simulink model "dbe_xaui"  (Simulink 7.0)
settings: Solver=ode45  StartTime=0.0  StopTime=1000  RelTol=1e-3
undefined symbols (not defined in the file or in its folder): iBOB, pfb_coeff_gen_calc
libraries: xbsIndex_r4 x1734, casper_library x124, xps_library x28, xbsReplacements_r4 x15, vlbi_lib x5, simulink x4, gavrt_library x1
(part 2 of 17)

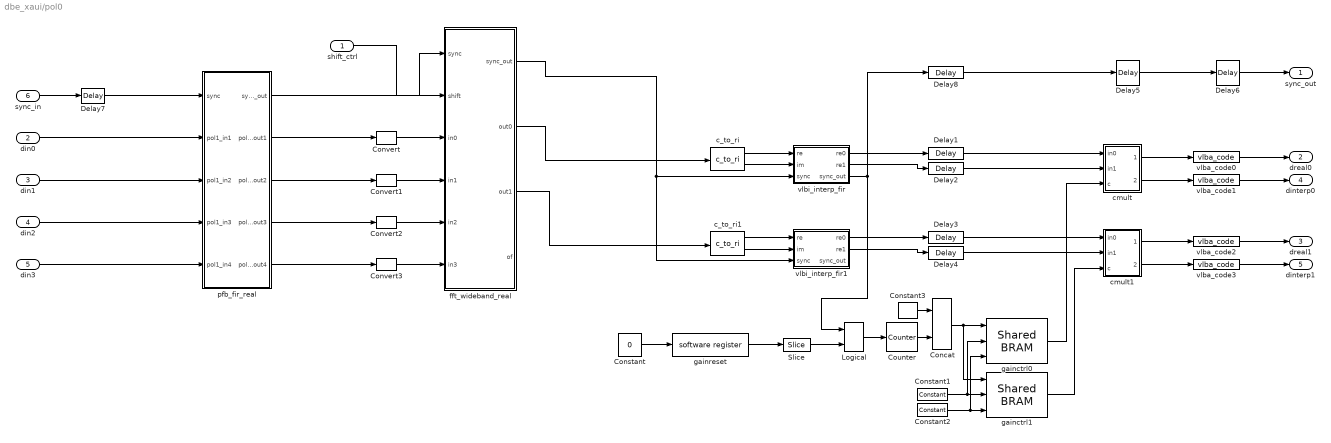
== system dbe_xaui/pol0 ==
  shift_ctrl : Inport  OutDataType=sfix(16)  OutScaling=2^0
  din0 : Inport  Port=2  OutDataType=sfix(16)  OutScaling=2^0
  din1 : Inport  Port=3  OutDataType=sfix(16)  OutScaling=2^0
  din2 : Inport  Port=4  OutDataType=sfix(16)  OutScaling=2^0
  din3 : Inport  Port=5  OutDataType=sfix(16)  OutScaling=2^0
  sync_in : Inport  Port=6  OutDataType=sfix(16)  OutScaling=2^0
  Concat : xbsIndex_r4/Concat  num_inputs=2  dbl_ovrd=off  block_type=concat
      settings: block_version=11.3
  Constant : Constant  Value=0  OutDataType=sfix(16)  OutScaling=2^0
  Constant1 : xbsIndex_r4/Constant  arith_type=Unsigned  const=0  n_bits=32  bin_pt=0  explicit_period=on  period=1  equ=P=C  opselect=C  inp2=PCIN>>17  opr=+  inp1=P  carry=CIN  dbl_ovrd=off  block_type=constant
      settings: block_version=11.3
  Constant2 : xbsIndex_r4/Constant  arith_type=Boolean  const=0  n_bits=1  bin_pt=0  explicit_period=on  period=1  equ=P=C  opselect=C  inp2=PCIN>>17  opr=+  inp1=P  carry=CIN  dbl_ovrd=off  block_type=constant
      settings: block_version=11.3
  Constant3 : xbsIndex_r4/Constant  arith_type=Unsigned  const=0  n_bits=8  bin_pt=0  explicit_period=on  period=1  equ=P=C  opselect=C  inp2=PCIN>>17  opr=+  inp1=P  carry=CIN  dbl_ovrd=off  block_type=constant
      settings: block_version=11.3
  Convert{,1..3} (4 alike) : xbsIndex_r4/Convert  arith_type="Signed  (2's comp)"  n_bits=18  bin_pt=17  quantization=Truncate  overflow=Wrap  latency=0  dbl_ovrd=off  pipeline=off  block_type=convert
      settings: xl_area=[0, 0, 0, 0, 0, 0, 0]  block_version=11.3
  Counter : xbsIndex_r4/Counter  cnt_type=Free Running  cnt_to=Inf  operation=Up  start_count=0  cnt_by_val=1  arith_type=Unsigned  n_bits=3  bin_pt=0  load_pin=off  rst=on  en=off  explicit_period=off  period=1  dbl_ovrd=off  use_behavioral_HDL=off  use_rpm=on  implementation=Fabric  block_type=counter
      settings: xl_area=[0, 0, 0, 0, 0, 0, 0]  block_version=11.3
  Delay1 : xbsIndex_r4/Delay  en=off  latency=1  dbl_ovrd=off  reg_retiming=off  block_type=delay
      settings: xl_area=[0, 0, 0, 0, 0, 0, 0]  block_version=11.3
  Delay{2..4,8} (4 alike) : xbsIndex_r4/Delay  en=off  latency=1  dbl_ovrd=off  reg_retiming=on  block_type=delay
      settings: xl_area=[0, 0, 0, 0, 0, 0, 0]  block_version=11.3
  Delay5 : xbsIndex_r4/Delay  en=off  latency=5  dbl_ovrd=off  reg_retiming=off  block_type=delay
      settings: xl_area=[0, 0, 0, 0, 0, 0, 0]  block_version=11.3
  Delay6 : xbsIndex_r4/Delay  en=off  latency=6  dbl_ovrd=off  reg_retiming=off  block_type=delay
      settings: xl_area=[0, 0, 0, 0, 0, 0, 0]  block_version=11.3
  Delay7 : xbsIndex_r4/Delay  en=off  latency=1  dbl_ovrd=off  reg_retiming=off  block_type=delay
      settings: xl_area=[0, 0, 0, 0, 0, 0, 0]  block_version=11.3
  Logical : xbsIndex_r4/Logical  logical_function=OR  inputs=2  en=off  latency=0  precision=Full  arith_type=Unsigned  n_bits=8  bin_pt=2  align_bp=on  dbl_ovrd=off  block_type=logical
      settings: xl_area=[0, 0, 0, 0, 0, 0, 0]  block_version=11.3
  Slice : xbsIndex_r4/Slice  nbits=1  boolean_output=on  mode=Lower Bit Location + Width  bit1=0  base1=MSB of Input  bit0=0  base0=LSB of Input  dbl_ovrd=off  block_type=slice
      settings: block_version=11.3
  c_to_ri : casper_library/Misc/c_to_ri  SystemSampleTime=-1  n_bits=18  bin_pt=17
      settings: AttributesFormatString=18_17 r/i
  c_to_ri1 : casper_library/Misc/c_to_ri  SystemSampleTime=-1  n_bits=18  bin_pt=17
      settings: AttributesFormatString=18_17 r/i
  cmult : Subsystem  mask{n_bits=18, mult_latency=3}
      mask prompts: n_bits "Bit Width"; mult_latency "Mult Latency"
  cmult1 : Subsystem  mask{n_bits=18, mult_latency=3}
      mask prompts: n_bits "Bit Width"; mult_latency "Mult Latency"
  fft_wideband_real : Subsystem  AncestorBlock=casper_library/FFTs/fft_wideband_real  mask(fft_wideband_real){FFTSize=5, input_bit_width=18, coeff_bit_width=18, n_inputs=2, quantization=Truncate, overflow=Wrap, add_latency=2, mult_latency=3, bram_latency=2, arch=Virtex5, opt_target=Multipliers, coeffs_bit_limit=8, delays_bit_limit=9, specify_mult=off, mult_spec=[2 2 2 2 2], dsp48_adders=off}
      mask prompts: FFTSize "Size of FFT: (2^?)"; input_bit_width "Input/Output Bit Width"; coeff_bit_width "Coefficient Bit Width"; n_inputs "Number of Simultaneous Inputs: (2^?)"; quantization "Quantization Behavior"; overflow "Overflow Behavior"; add_latency "Add Latency"; mult_latency "Mult Latency"; bram_latency "BRAM Latency"; arch "Architecture"; opt_target "Optimisation Target"; coeffs_bit_limit "Number bits above which to store stage's coefficients in BRAM (2^? bits)"; delays_bit_limit "Number bits above which to store stage's delays in BRAM (2^? bits)"; specify_mult "Specify multiplier use (behavioral HDL if not specified)"; mult_spec "Multiplier specification (0=core, 1=embedded, 2=behavioural) (left=1st stage)"; dsp48_adders "Use DSP48s for adders"
      mask init:
      | fft_wideband_real_init(gcb,...
      |     'FFTSize', FFTSize,...
      |     'input_bit_width', input_bit_width,...
      |     'coeff_bit_width', coeff_bit_width,...
      |     'n_inputs', n_inputs,...
      |     'quantization', quantization,...
      |     'overflow', overflow,...
      |     'add_latency', add_latency,...
      |     'mult_latency', mult_latency,...
      |     'bram_latency', bram_latency,...
      |     'arch', arch, ...
      |     'opt_target', opt_target, ...
      |     'coeffs_bit_limit', coeffs_bit_limit, ...
      |     'delays_bit_limit', delays_bit_limit, ...    
      |     'specify_mult', specify_mult,...
      |     'mult_spec', mult_spec, ...
      |     'dsp48_adders', dsp48_adders);
      settings: AttributesFormatString=FFTSize=5
      mask Help: eval('xlWeb([getenv(''MLIB_ROOT''), ''\doc\htmlblocks\sp_fft_wideband_real\sp_fft_wideband_real.html''])')
      mask StyleString: edit,edit,edit,edit,popup(Truncate|Round  (unbiased: +/- Inf)|Round  (unbiased: Even Values)),popup(Wrap|Saturate|Error),edit,edit,edit,popup(Virtex2Pro|Virtex5),popup(Logic|Multipliers),edit,edit,checkbox,edit,checkbox
      mask CallbackString:
      | % fft_size = eval(get_param(gcb, 'FFTSize'));
      | % old_vec = eval(get_param(gcb, 'mult_spec'));
      | 
      | % vec = 2.*ones(1,fft_size);
      | % old_size = length(old_vec);
      | 
      | % if(old_size <= fft_size),
      | %    size = old_size;
      | % else,
      | %     size = fft_size;
      | % end
      | 
      | % for n = 1:size, vec(n) = old_vec(n); end
      | % set_param(gcb, 'mult_spec', mat2str(vec));|||||||||||||specify_mult = get_param(gcb,'specify_mult');
      | visibs = get_param(gcb, 'MaskVisibilities');
      | enables = get_param(gcb, 'MaskEnables');
      | enables{15} = 'on';
      | if( strcmp(specify_mult,'off')), visibs{15} = 'off';
      | else, visibs{15} = 'on';
      | end
      | set_param(gcb, 'MaskEnables', enables);
      | set_param(gcb, 'MaskVisibilities', visibs);
      | |% vec = eval( get_param(gcb, 'mult_spec'));
      | % vec_size = length(vec);
      | % fft_size = eval( get_param(gcb, 'FFTSize'));
      | % new_vec = 2.*ones(1, fft_size);
      | 
      | % if(vec_size <= fft_size), size = vec_size;
      | % else size = fft_size;
      | % end
      | 
      | % for n=1:size
      | %     new_vec(n) = vec(n);
      | % end
      | % set_param(gcb, 'mult_spec', mat2str(new_vec));|
  gainctrl0 : xps_library/Shared BRAM  Tag=xps:bram  SystemSampleTime=-1  arith_type="Signed  (2's comp)"  addr_width=11  data_width=32  data_bin_pt=0  init_vals=[0 0 100 0 zeros(1,2^11-4)]  sample_rate=1
      link override convert_din: arith_type="Signed  (2's comp)"
      link override dbe_xaui_pol0_gainctrl0_data_out: arith_type="Signed  (2's comp)"
      link override mem/sim_data_in: arith_type="Signed  (2's comp)"
      link override vlbipfb2_m3_ibob_pol0_gainctrl0_data_out: arith_type="Signed  (2's comp)"
      link override vlbipfb2_m3_roach_pol0_gainctrl0_data_out: arith_type="Signed  (2's comp)"
  gainctrl1 : xps_library/Shared BRAM  Tag=xps:bram  SystemSampleTime=-1  arith_type="Signed  (2's comp)"  addr_width=11  data_width=32  data_bin_pt=0  init_vals=zeros(1,2^11)  sample_rate=1
      link override convert_din: arith_type="Signed  (2's comp)"
      link override dbe_xaui_pol0_gainctrl1_data_out: arith_type="Signed  (2's comp)"
      link override mem/sim_data_in: arith_type="Signed  (2's comp)"
      link override vlbipfb2_m3_ibob_pol0_gainctrl1_data_out: arith_type="Signed  (2's comp)"
      link override vlbipfb2_m3_roach_pol0_gainctrl1_data_out: arith_type="Signed  (2's comp)"
  gainreset : xps_library/software register  Tag=xps:sw_reg  SystemSampleTime=-1  io_dir=From Processor  arith_type=Unsigned  bitwidth=32  bin_pt=0  sample_period=1  gw_name=dbe_xaui_pol0_gainreset_user_data_out
      link override dbe_xaui_pol0_gainreset_user_data_out: arith_type=Unsigned  n_bits=32  bin_pt=0  period=1
      link override vlbipfb2_m3_ibob_pol0_gainreset_user_data_out: arith_type=Unsigned  n_bits=32  bin_pt=0  period=1
      link override vlbipfb2_m3_roach_pol0_gainreset_user_data_out: arith_type=Unsigned  n_bits=32  bin_pt=0  period=1
  pfb_fir_real : Subsystem  AncestorBlock=casper_library/PFBs/pfb_fir_real  mask(pfb_fir_real){PFBSize=5, TotalTaps=8, WindowType=hamming, n_inputs=2, MakeBiplex=0, BitWidthIn=8, BitWidthOut=8, CoeffBitWidth=8, CoeffDistMem=0, add_latency=2, mult_latency=3, bram_latency=2, quantization=Truncate, fwidth=1, specify_mult=off, mult_spec=[0 1 2 0 1 1 1 1]}
      mask prompts: PFBSize "Size of PFB: (2^? pnts)"; TotalTaps "Total Number of Taps:"; WindowType "Windowing Function:"; n_inputs "Number of Simultaneous Inputs: (2^?)"; MakeBiplex "Make Biplex"; BitWidthIn "Input Bitwidth:"; BitWidthOut "Output Bitwidth:"; CoeffBitWidth "Coefficient Bitwidth:"; CoeffDistMem "Use Distributed Memory for Coeffs"; add_latency "Add Latency"; mult_latency "Mult Latency"; bram_latency "BRAM Latency"; quantization "Quantization Behavior"; fwidth "Bin Width Scaling (normal=1)"; specify_mult "Specify multiplier use (embedded if not specified)"; mult_spec "Multiplier specification (0=core, 1=embedded, 2=behavioural) (left=1st tap)"
      mask init:
      | pfb_fir_real_init(gcb,...
      |     'PFBSize', PFBSize,...
      |     'TotalTaps', TotalTaps,...
      |     'WindowType', WindowType,...
      |     'n_inputs', n_inputs,...
      |     'MakeBiplex', MakeBiplex,...
      |     'BitWidthIn', BitWidthIn,...
      |     'BitWidthOut', BitWidthOut,...
      |     'CoeffBitWidth', CoeffBitWidth,...
      |     'CoeffDistMem', CoeffDistMem,...
      |     'add_latency', add_latency,...
      |     'mult_latency', mult_latency,...
      |     'bram_latency', bram_latency,...
      |     'quantization', quantization,...
      |     'fwidth', fwidth,...
      |     'specify_mult', specify_mult,...
      |     'mult_spec', mult_spec);
      settings: AttributesFormatString=taps=8, add_latency=2
      mask Help: eval('xlWeb([getenv(''MLIB_ROOT''), ''\doc\htmlblocks\sp_pfb_fir_real\sp_pfb_fir_real.html''])')
      mask StyleString: edit,edit,popup(bartlett|barthannwin|blackman|blackmanharris|bohamwin|chebwin|flattopwin|gausswin|hamming|hann|kaiser|nuttallwin|parzenwin|rectwin|tukeywin|triang|userwindow),edit,edit,edit,edit,edit,edit,edit,edit,edit,popup(Truncate|Round  (unbiased: +/- Inf)|Round  (unbiased: Even Values)),edit,checkbox,edit
      mask CallbackString:
      | |num_taps = eval(get_param(gcb, 'TotalTaps'));
      | old_vec = eval(get_param(gcb, 'mult_spec'));
      | 
      | vec = ones(1,num_taps);
      | old_size = length(old_vec);
      | 
      | if(old_size <= num_taps),
      |     size = old_size;
      | else,
      |     size = num_taps;
      | end
      | 
      | for n = 1:size, vec(n) = old_vec(n); end
      | set_param(gcb, 'mult_spec', mat2str(vec));|||||||||||||specify_mult = get_param(gcb,'specify_mult');
      | if( strcmp(specify_mult,'off')),
      |     set_param(gcb, 'MaskEnables', {'on','on','on','on','on','on','on','on','on','on','on','on','on','on','on','off'});
      | else
      |     set_param(gcb, 'MaskEnables', {'on','on','on','on','on','on','on','on','on','on','on','on','on','on','on','on'});
      | end
      | 
      | |vec = eval( get_param(gcb, 'mult_spec'));
      | vec_size = length(vec);
      | num_taps = eval( get_param(gcb, 'TotalTaps'));
      | new_vec = ones(1, num_taps);
      | 
      | if(vec_size <= num_taps), size = vec_size;
      | else size = num_taps;
      | end
      | 
      | for n=1:size
      |     new_vec(n) = vec(n);
      | end
      | set_param(gcb, 'mult_spec', mat2str(new_vec));
  vlba_code{0..3} (4 alike) : vlbi_lib/vlba_code  SystemSampleTime=-1  bitwidth=18
  vlbi_interp_fir : Subsystem  mask{tap_delay=8, n_bits=18, add_latency=3, mult_latency=3, bram_latency=2}
      mask prompts: tap_delay "Delay between Taps"; n_bits "Bit Width"; add_latency "Add Latency"; mult_latency "Mult Latency"; bram_latency "BRAM Latency"
      mask init:
      | for i=0:4,
      |     x = pi*(2*i+1)/2;
      |     coeff(i+1) = sin(x)/x;
      | end
      | coeff = coeff / (2*sum(coeff));
  vlbi_interp_fir1 : Subsystem  mask{tap_delay=8, n_bits=18, add_latency=3, mult_latency=3, bram_latency=2}
      mask prompts: tap_delay "Delay between Taps"; n_bits "Bit Width"; add_latency "Add Latency"; mult_latency "Mult Latency"; bram_latency "BRAM Latency"
      mask init:
      | for i=0:4,
      |     x = pi*(2*i+1)/2;
      |     coeff(i+1) = sin(x)/x;
      | end
      | coeff = coeff / (2*sum(coeff));
  sync_out : Outport  OutDataType=sfix(16)  OutScaling=2^0
  dreal0 : Outport  Port=2  OutDataType=sfix(16)  OutScaling=2^0
  dreal1 : Outport  Port=3  OutDataType=sfix(16)  OutScaling=2^0
  dinterp0 : Outport  Port=4  OutDataType=sfix(16)  OutScaling=2^0
  dinterp1 : Outport  Port=5  OutDataType=sfix(16)  OutScaling=2^0
  wires:
  sync_in/1 -> Delay7/1
  din0/1 -> pfb_fir_real/2(pol1_in1)
  din2/1 -> pfb_fir_real/4(pol1_in3)
  din3/1 -> pfb_fir_real/5(pol1_in4)
  c_to_ri/1 -> vlbi_interp_fir/1(re)
  c_to_ri/2 -> vlbi_interp_fir/2(im)
  c_to_ri1/1 -> vlbi_interp_fir1/1(re)
  c_to_ri1/2 -> vlbi_interp_fir1/2(im)
  vlbi_interp_fir/1(re0) -> Delay1/1
  vlbi_interp_fir/2(re1) -> Delay2/1
  vlbi_interp_fir1/1(re0) -> Delay3/1
  vlbi_interp_fir1/2(re1) -> Delay4/1
  vlbi_interp_fir/3(sync_out) -> Delay8/1, Logical/1
  Delay6/1 -> sync_out/1
  vlba_code0/1 -> dreal0/1
  vlba_code1/1 -> dinterp0/1
  vlba_code2/1 -> dreal1/1
  vlba_code3/1 -> dinterp1/1
  din1/1 -> pfb_fir_real/3(pol1_in2)
  shift_ctrl/1 -> fft_wideband_real/2(shift)
  Delay8/1 -> Delay5/1
  Constant3/1 -> Concat/1
  Counter/1 -> Concat/2
  Concat/1 -> gainctrl0/1, gainctrl1/1
  Constant1/1 -> gainctrl0/2, gainctrl1/2
  Constant2/1 -> gainctrl1/3, gainctrl0/3
  Delay5/1 -> Delay6/1
  Delay1/1 -> cmult/1(in0)
  Delay2/1 -> cmult/2(in1)
  Delay3/1 -> cmult1/1(in0)
  Delay4/1 -> cmult1/2(in1)
  gainctrl0/1 -> cmult/3(c)
  gainctrl1/1 -> cmult1/3(c)
  cmult/1(out0) -> vlba_code0/1
  cmult/2(out1) -> vlba_code1/1
  cmult1/1(out0) -> vlba_code2/1
  cmult1/2(out1) -> vlba_code3/1
  fft_wideband_real/3(out1) -> c_to_ri1/1
  Delay7/1 -> pfb_fir_real/1(sync)
  pfb_fir_real/5(pol1_out4) -> Convert3/1
  pfb_fir_real/4(pol1_out3) -> Convert2/1
  pfb_fir_real/3(pol1_out2) -> Convert1/1
  pfb_fir_real/1(sync_out) -> fft_wideband_real/1(sync)
  Convert/1 -> fft_wideband_real/3(in0)
  Convert1/1 -> fft_wideband_real/4(in1)
  Convert2/1 -> fft_wideband_real/5(in2)
  Convert3/1 -> fft_wideband_real/6(in3)
  Constant/1 -> gainreset/1
  Logical/1 -> Counter/1
  gainreset/1 -> Slice/1
  Slice/1 -> Logical/2
  fft_wideband_real/1(sync_out) -> vlbi_interp_fir/3(sync), vlbi_interp_fir1/3(sync)
  fft_wideband_real/2(out0) -> c_to_ri/1
  pfb_fir_real/2(pol1_out1) -> Convert/1

== system dbe_xaui/twobitsimscope ==
  In1 : Inport  OutDataType=sfix(16)  OutScaling=2^0
  In2 : Inport  Port=2  OutDataType=sfix(16)  OutScaling=2^0
  Concat3 : xbsIndex_r4/Concat  num_inputs=2  dbl_ovrd=off  block_type=concat
      settings: block_version=11.3
  Convert : xbsIndex_r4/Convert  arith_type=Boolean  n_bits=8  bin_pt=6  quantization=Truncate  overflow=Wrap  latency=0  dbl_ovrd=off  pipeline=off  block_type=convert
      settings: xl_area=[0, 0, 0, 0, 0, 0, 0]  block_version=11.3
  Delay : xbsIndex_r4/Delay  en=off  latency=1  dbl_ovrd=off  reg_retiming=off  block_type=delay
      settings: xl_area=[0, 0, 0, 0, 0, 0, 0]  block_version=11.3
  Disregard Subsystem : xbsIndex_r4/Disregard Subsystem  Tag=discardX  block_type=disregard
      settings: block_version=11.3
  Expression : xbsIndex_r4/Expression  expression=a & ~b  align_bp=on  en=off  latency=0  precision=Full  arith_type=Unsigned  n_bits=8  bin_pt=2  dbl_ovrd=off  block_type=expr
      settings: xl_area=[0, 0, 0, 0, 0, 0, 0]  block_version=11.3
  Gateway Out3 : xbsIndex_r4/Gateway Out  hdl_port=off  timing_constraint=None  locs_specified=off  LOCs={}  block_type=gatewayout
      settings: infoedit="Gateway out block.  Converts Xilinx fixed point inputs into ouputs of type Simulink integer, double, or fixed point.<br><br>Hardware notes:  In hardware these blocks become top level output ports or are discarded, depending on how they are configured."  xl_area=[0, 0, 0, 0, 0, 0, 0]  block_version=11.3
  Gateway Out4 : xbsIndex_r4/Gateway Out  hdl_port=off  timing_constraint=None  locs_specified=off  LOCs={}  block_type=gatewayout
      settings: infoedit="Gateway out block.  Converts Xilinx fixed point inputs into ouputs of type Simulink integer, double, or fixed point.<br><br>Hardware notes:  In hardware these blocks become top level output ports or are discarded, depending on how they are configured."  xl_area=[0, 0, 0, 0, 0, 0, 0]  block_version=11.3
  Gateway Out5 : xbsIndex_r4/Gateway Out  hdl_port=off  timing_constraint=None  locs_specified=off  LOCs={}  block_type=gatewayout
      settings: infoedit="Gateway out block.  Converts Xilinx fixed point inputs into ouputs of type Simulink integer, double, or fixed point.<br><br>Hardware notes:  In hardware these blocks become top level output ports or are discarded, depending on how they are configured."  xl_area=[0, 0, 0, 0, 0, 0, 0]  block_version=11.3
  Register1 : xbsIndex_r4/Register  init=0  rst=off  en=on  dbl_ovrd=off  block_type=register
      settings: xl_area=[0, 0, 0, 0, 0, 0, 0]  block_version=11.3
  Register2 : xbsIndex_r4/Register  init=0  rst=off  en=on  dbl_ovrd=off  block_type=register
      settings: xl_area=[0, 0, 0, 0, 0, 0, 0]  block_version=11.3
  Scope3 : Scope  NumInputPorts=3
      settings: YMin=-5~-5~-5  YMax=5~5~5  SaveName=ScopeData7  MaxDataPoints=10000
  Slice : xbsIndex_r4/Slice  nbits=1  boolean_output=off  mode=Lower Bit Location + Width  bit1=9  base1=MSB of Input  bit0=9  base0=LSB of Input  dbl_ovrd=off  block_type=slice
      settings: block_version=11.3
  Slice1 : xbsIndex_r4/Slice  nbits=1  boolean_output=off  mode=Lower Bit Location + Width  bit1=-9  base1=MSB of Input  bit0=8  base0=LSB of Input  dbl_ovrd=off  block_type=slice
      settings: block_version=11.3
  wires:
  Convert/1 -> Register2/2, Register1/2
  Expression/1 -> Convert/1
  Slice1/1 -> Register2/1
  Register1/1 -> Gateway Out3/1, Concat3/1
  Slice/1 -> Register1/1
  Delay/1 -> Expression/2
  Concat3/1 -> Gateway Out5/1
  Gateway Out5/1 -> Scope3/3
  Register2/1 -> Gateway Out4/1, Concat3/2
  Gateway Out4/1 -> Scope3/2
  Gateway Out3/1 -> Scope3/1
  In1/1 -> Slice/1, Slice1/1
  In2/1 -> Delay/1, Expression/1

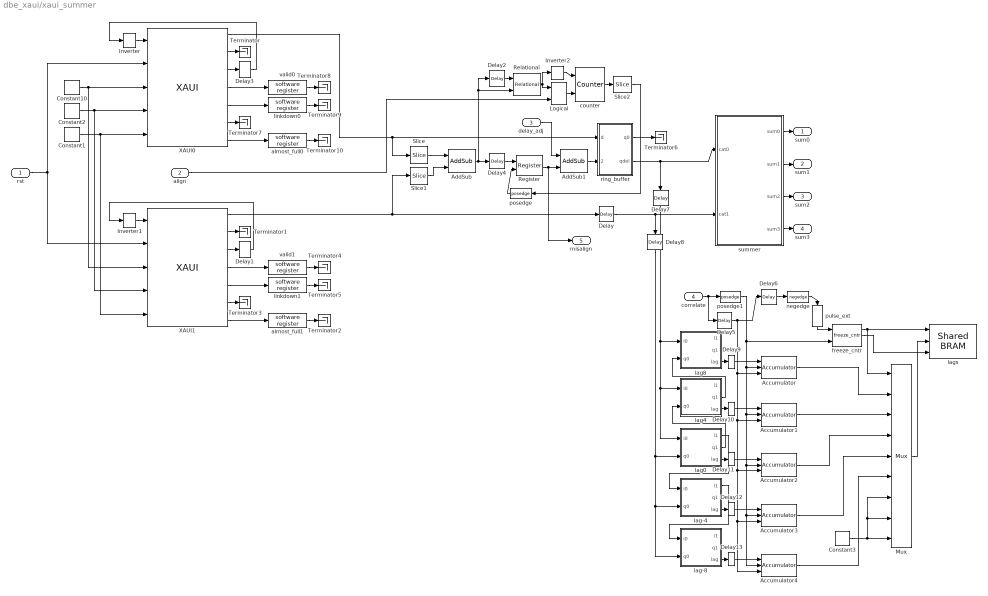
== system dbe_xaui/xaui_summer ==
  rst : Inport  OutDataType=sfix(16)  OutScaling=2^0
  align : Inport  Port=2  OutDataType=sfix(16)  OutScaling=2^0
  delay_adj : Inport  Port=3  OutDataType=sfix(16)  OutScaling=2^0
  correlate : Inport  Port=4  OutDataType=sfix(16)  OutScaling=2^0
  Accumulator{,1..4} (5 alike) : xbsIndex_r4/Accumulator  operation=Add  n_bits=32  overflow=Saturate  scale=1  rst=on  hasbypass=off  en=on  dbl_ovrd=off  use_behavioral_HDL=on  implementation=Fabric  block_type=accum
      settings: infoedit="Adder or subtractor-based accumulator.   Output type and binary point position match the input.<P><P>Hardware notes: When \"Reinitialize with input 'b' on reset\" is selected, the accumulator is forced to run at the system rate even if the input 'b' is running at a slower rate."  block_version=10.1.3
  AddSub : xbsIndex_r4/AddSub  mode=Subtraction  use_carryin=off  use_carryout=off  en=off  latency=2  precision=Full  arith_type=Unsigned  n_bits=16  bin_pt=14  quantization=Truncate  overflow=Wrap  dbl_ovrd=off  use_behavioral_HDL=off  pipelined=off  use_rpm=on  hw_selection=Fabric  block_type=addsub
      settings: block_version=10.1.3
  AddSub1 : xbsIndex_r4/AddSub  mode=Addition  use_carryin=off  use_carryout=off  en=off  latency=2  precision=User Defined  arith_type=Unsigned  n_bits=4  bin_pt=0  quantization=Truncate  overflow=Wrap  dbl_ovrd=off  use_behavioral_HDL=off  pipelined=off  use_rpm=on  hw_selection=Fabric  block_type=addsub
      settings: block_version=10.1.3
  Constant1 : xbsIndex_r4/Constant  arith_type=Boolean  const=0  n_bits=4  bin_pt=0  explicit_period=on  period=1  equ=P=C  opselect=C  inp2=PCIN>>17  opr=+  inp1=P  carry=CIN  dbl_ovrd=off  block_type=constant
      settings: block_version=11.3
  Constant10 : xbsIndex_r4/Constant  arith_type=Unsigned  const=2^4 -1  n_bits=32  bin_pt=0  explicit_period=on  period=1  equ=P=C  opselect=C  inp2=PCIN>>17  opr=+  inp1=P  carry=CIN  dbl_ovrd=off  block_type=constant
      settings: block_version=11.3
  Constant2 : xbsIndex_r4/Constant  arith_type=Unsigned  const=0  n_bits=4  bin_pt=0  explicit_period=on  period=1  equ=P=C  opselect=C  inp2=PCIN>>17  opr=+  inp1=P  carry=CIN  dbl_ovrd=off  block_type=constant
      settings: block_version=11.3
  Constant3 : xbsIndex_r4/Constant  arith_type=Unsigned  const=2^32 - 1  n_bits=32  bin_pt=0  explicit_period=on  period=1  equ=P=C  opselect=C  inp2=PCIN>>17  opr=+  inp1=P  carry=CIN  dbl_ovrd=off  block_type=constant
      settings: block_version=11.3
  Delay : xbsIndex_r4/Delay  en=off  latency=8  dbl_ovrd=off  reg_retiming=on  block_type=delay
  Delay1 : xbsIndex_r4/Delay  en=off  latency=32  dbl_ovrd=off  reg_retiming=off  block_type=delay
      settings: block_version=11.3
  Delay{4..6,9..13} (8 alike) : xbsIndex_r4/Delay  en=off  latency=2  dbl_ovrd=off  reg_retiming=on  block_type=delay
  Delay2 : xbsIndex_r4/Delay  en=off  latency=1  dbl_ovrd=off  reg_retiming=on  block_type=delay
  Delay3 : xbsIndex_r4/Delay  en=off  latency=32  dbl_ovrd=off  reg_retiming=off  block_type=delay
      settings: block_version=11.3
  Delay7 : xbsIndex_r4/Delay  Orientation=down  en=off  latency=30  dbl_ovrd=off  reg_retiming=on  block_type=delay
  Delay8 : xbsIndex_r4/Delay  Orientation=down  en=off  latency=30  dbl_ovrd=off  reg_retiming=on  block_type=delay
  Inverter : xbsIndex_r4/Inverter  en=off  latency=0  dbl_ovrd=off  block_type=inv
      settings: block_version=11.3
  Inverter1 : xbsIndex_r4/Inverter  en=off  latency=0  dbl_ovrd=off  block_type=inv
      settings: block_version=11.3
  Inverter2 : xbsIndex_r4/Inverter  en=off  latency=0  dbl_ovrd=off  block_type=inv
  Logical : xbsIndex_r4/Logical  logical_function=AND  inputs=2  en=off  latency=0  precision=Full  arith_type=Unsigned  n_bits=16  bin_pt=0  align_bp=on  dbl_ovrd=off  block_type=logical
  Mux : xbsIndex_r4/Mux  inputs=8  en=off  latency=0  precision=Full  arith_type=Unsigned  n_bits=16  bin_pt=14  quantization=Truncate  overflow=Wrap  dbl_ovrd=off  block_type=mux
  Register : xbsIndex_r4/Register  init=0  rst=off  en=on  dbl_ovrd=off  block_type=register
  Relational : xbsIndex_r4/Relational  mode=a=b  en=off  latency=1  dbl_ovrd=off  block_type=relational
      settings: block_version=10.1.3
  Slice : xbsIndex_r4/Slice  nbits=4  boolean_output=off  mode=Lower Bit Location + Width  bit1=0  base1=MSB of Input  bit0=0  base0=LSB of Input  dbl_ovrd=off  block_type=slice
      settings: infoedit="Extracts a given range of bits from each input sample and presents it at the output.  The output type is ordinarily unsigned with binary point at zero, but can be Boolean when the slice is one bit wide.<P><P>Hardware notes: In hardware this block costs nothing."  block_version=10.1.3
  Slice1 : xbsIndex_r4/Slice  nbits=4  boolean_output=off  mode=Lower Bit Location + Width  bit1=0  base1=MSB of Input  bit0=0  base0=LSB of Input  dbl_ovrd=off  block_type=slice
      settings: infoedit="Extracts a given range of bits from each input sample and presents it at the output.  The output type is ordinarily unsigned with binary point at zero, but can be Boolean when the slice is one bit wide.<P><P>Hardware notes: In hardware this block costs nothing."  block_version=10.1.3
  Slice2 : xbsIndex_r4/Slice  nbits=1  boolean_output=on  mode=Upper Bit Location + Width  bit1=0  base1=MSB of Input  bit0=0  base0=LSB of Input  dbl_ovrd=off  block_type=slice
      settings: block_version=10.1.3
  Terminator{,1..10} (11 alike) : Terminator
  XAUI0 : xps_library/XAUI  Tag=xps:xaui  SystemSampleTime=-1  demux=2  port=iBOB:0  open_phy=off  show_param=off  pre_emph=3  swing=800
      link override convert_tx_data: n_bits=32
      link override convert_tx_outofband: n_bits=4
      link override dbe_xaui_xaui_summer_XAUI0_rx_data: n_bits=32
      link override dbe_xaui_xaui_summer_XAUI0_rx_outofband: n_bits=4
      link override xaui_XAUI_RECV_rx_data: n_bits=32
      link override xaui_XAUI_RECV_rx_outofband: n_bits=4
  XAUI1 : xps_library/XAUI  Tag=xps:xaui  SystemSampleTime=-1  demux=2  port=iBOB:1  open_phy=off  show_param=off  pre_emph=3  swing=800
      link override convert_tx_data: n_bits=32
      link override convert_tx_outofband: n_bits=4
      link override dbe_xaui_xaui_summer_XAUI1_rx_data: n_bits=32
      link override dbe_xaui_xaui_summer_XAUI1_rx_outofband: n_bits=4
      link override xaui_XAUI_RECV_rx_data: n_bits=32
      link override xaui_XAUI_RECV_rx_outofband: n_bits=4
  almost_full0 : xps_library/software register  Tag=xps:sw_reg  SystemSampleTime=-1  io_dir=To Processor  arith_type=Unsigned  bitwidth=32  bin_pt=0  sample_period=1  gw_name=dbe_xaui_xaui_summer_almost_full0_user_data_in
      link override convert: arith_type=Unsigned  n_bits=32  bin_pt=0
  almost_full1 : xps_library/software register  Tag=xps:sw_reg  SystemSampleTime=-1  io_dir=To Processor  arith_type=Unsigned  bitwidth=32  bin_pt=0  sample_period=1  gw_name=dbe_xaui_xaui_summer_almost_full1_user_data_in
      link override convert: arith_type=Unsigned  n_bits=32  bin_pt=0
  counter : xbsIndex_r4/Counter  cnt_type=Free Running  cnt_to=Inf  operation=Up  start_count=0  cnt_by_val=1  arith_type=Unsigned  n_bits=4  bin_pt=0  load_pin=off  rst=on  en=on  explicit_period=on  period=1  dbl_ovrd=off  use_behavioral_HDL=off  use_rpm=off  implementation=Fabric  block_type=counter
  freeze_cntr : casper_library/Misc/freeze_cntr  SystemSampleTime=-1  CounterBits=3
  lag-4 : Subsystem
  lag-8 : Subsystem
  lag0 : Subsystem
  lag4 : Subsystem
  lag8 : Subsystem
  lags : xps_library/Shared BRAM  Tag=xps:bram  SystemSampleTime=-1  arith_type="Signed  (2's comp)"  addr_width=11  data_width=32  data_bin_pt=0  init_vals=[1:2^11]  sample_rate=1
      link override convert_din: arith_type="Signed  (2's comp)"
      link override dbe_xaui_xaui_summer_lags_data_out: arith_type="Signed  (2's comp)"
      link override mem/sim_data_in: arith_type="Signed  (2's comp)"
  linkdown0 : xps_library/software register  Tag=xps:sw_reg  SystemSampleTime=-1  io_dir=To Processor  arith_type=Unsigned  bitwidth=32  bin_pt=0  sample_period=1  gw_name=dbe_xaui_xaui_summer_linkdown0_user_data_in
      link override convert: arith_type=Unsigned  n_bits=32  bin_pt=0
  linkdown1 : xps_library/software register  Tag=xps:sw_reg  SystemSampleTime=-1  io_dir=To Processor  arith_type=Unsigned  bitwidth=32  bin_pt=0  sample_period=1  gw_name=dbe_xaui_xaui_summer_linkdown1_user_data_in
      link override convert: arith_type=Unsigned  n_bits=32  bin_pt=0
  negedge : casper_library/Misc/negedge  Tag=Rising Edge Detector  SystemSampleTime=-1
  posedge : casper_library/Misc/posedge  Tag=Rising Edge Detector  Orientation=left  SystemSampleTime=-1
  posedge1 : casper_library/Misc/posedge  Tag=Rising Edge Detector  SystemSampleTime=-1
  pulse_ext : casper_library/Misc/pulse_ext  Orientation=down  SystemSampleTime=-1  pulse_len=8
  ring_buffer : Subsystem
  summer : Subsystem
  valid0 : xps_library/software register  Tag=xps:sw_reg  SystemSampleTime=-1  io_dir=To Processor  arith_type=Unsigned  bitwidth=32  bin_pt=0  sample_period=1  gw_name=dbe_xaui_xaui_summer_valid0_user_data_in
      link override convert: arith_type=Unsigned  n_bits=32  bin_pt=0
  valid1 : xps_library/software register  Tag=xps:sw_reg  SystemSampleTime=-1  io_dir=To Processor  arith_type=Unsigned  bitwidth=32  bin_pt=0  sample_period=1  gw_name=dbe_xaui_xaui_summer_valid1_user_data_in
      link override convert: arith_type=Unsigned  n_bits=32  bin_pt=0
  sum0 : Outport
  sum1 : Outport  Port=2
  sum2 : Outport  Port=3
  sum3 : Outport  Port=4
  misalign : Outport  Port=5
  wires:
  XAUI0/2 -> Terminator/1
  Constant2/1 -> XAUI0/4, XAUI1/4
  XAUI0/3 -> Delay3/1
  Delay3/1 -> Inverter/1
  Inverter/1 -> XAUI0/1
  XAUI0/6 -> Terminator7/1
  Constant10/1 -> XAUI0/3, XAUI1/3
  rst/1 -> XAUI0/2, XAUI1/2
  XAUI0/4 -> valid0/1
  XAUI0/5 -> linkdown0/1
  valid0/1 -> Terminator8/1
  linkdown0/1 -> Terminator9/1
  almost_full0/1 -> Terminator10/1
  XAUI0/7 -> almost_full0/1
  XAUI1/2 -> Terminator1/1
  XAUI1/3 -> Delay1/1
  Inverter1/1 -> XAUI1/1
  XAUI1/6 -> Terminator3/1
  XAUI1/4 -> valid1/1
  XAUI1/5 -> linkdown1/1
  valid1/1 -> Terminator4/1
  linkdown1/1 -> Terminator5/1
  almost_full1/1 -> Terminator2/1
  XAUI1/7 -> almost_full1/1
  Delay1/1 -> Inverter1/1
  summer/1(sum0) -> sum0/1
  summer/2(sum1) -> sum1/1
  summer/3(sum2) -> sum2/1
  summer/4(sum3) -> sum3/1
  align/1 -> Logical/2
  Slice/1 -> AddSub/1
  Slice1/1 -> AddSub/2
  AddSub/1 -> Relational/2, Delay2/1, Delay4/1
  Delay2/1 -> Relational/1
  AddSub1/1 -> ring_buffer/2(delay)
  Register/1 -> AddSub1/2, misalign/1
  Logical/1 -> counter/2
  Relational/1 -> Inverter2/1, Logical/1
  Inverter2/1 -> counter/1
  XAUI0/1 -> Slice/1, ring_buffer/1(d)
  counter/1 -> Slice2/1
  Slice2/1 -> posedge/1
  Delay4/1 -> Register/1
  XAUI1/1 -> Delay/1, Slice1/1
  posedge/1 -> Register/2
  Delay/1 -> summer/2(cat1), Delay8/1
  delay_adj/1 -> AddSub1/1
  ring_buffer/2(qdel) -> summer/1(cat0), Delay7/1
  ring_buffer/1(q0) -> Terminator6/1
  lag0/2(q1) -> lag4/2(q0)
  lag4/2(q1) -> lag8/2(q0)
  lag0/1(i1) -> lag-4/1(i0)
  lag-4/1(i1) -> lag-8/1(i0)
  lag8/3(lag) -> Delay9/1
  lag4/3(lag) -> Delay10/1
  lag0/3(lag) -> Delay11/1
  lag-4/3(lag) -> Delay12/1
  lag-8/3(lag) -> Delay13/1
  correlate/1 -> Delay5/1, posedge1/1
  Delay5/1 -> Accumulator/3, Accumulator1/3, Accumulator2/3, Accumulator3/3, Accumulator4/3, Delay6/1
  posedge1/1 -> Accumulator/2, Accumulator1/2, Accumulator2/2, Accumulator3/2, Accumulator4/2, freeze_cntr/2
  Delay6/1 -> negedge/1
  negedge/1 -> pulse_ext/1
  pulse_ext/1 -> freeze_cntr/1
  freeze_cntr/1 -> lags/1, Mux/1
  freeze_cntr/2 -> lags/3
  Mux/1 -> lags/2
  Accumulator/1 -> Mux/2
  Accumulator1/1 -> Mux/3
  Accumulator2/1 -> Mux/4
  Accumulator3/1 -> Mux/5
  Accumulator4/1 -> Mux/6
  Constant1/1 -> XAUI0/5, XAUI1/5
  Constant3/1 -> Mux/9, Mux/8, Mux/7
  Delay7/1 -> lag4/1(i0), lag0/1(i0), lag8/1(i0)
  Delay8/1 -> lag-4/2(q0), lag-8/2(q0), lag0/2(q0)
  Delay9/1 -> Accumulator/1
  Delay10/1 -> Accumulator1/1
  Delay11/1 -> Accumulator2/1
  Delay12/1 -> Accumulator3/1
  Delay13/1 -> Accumulator4/1

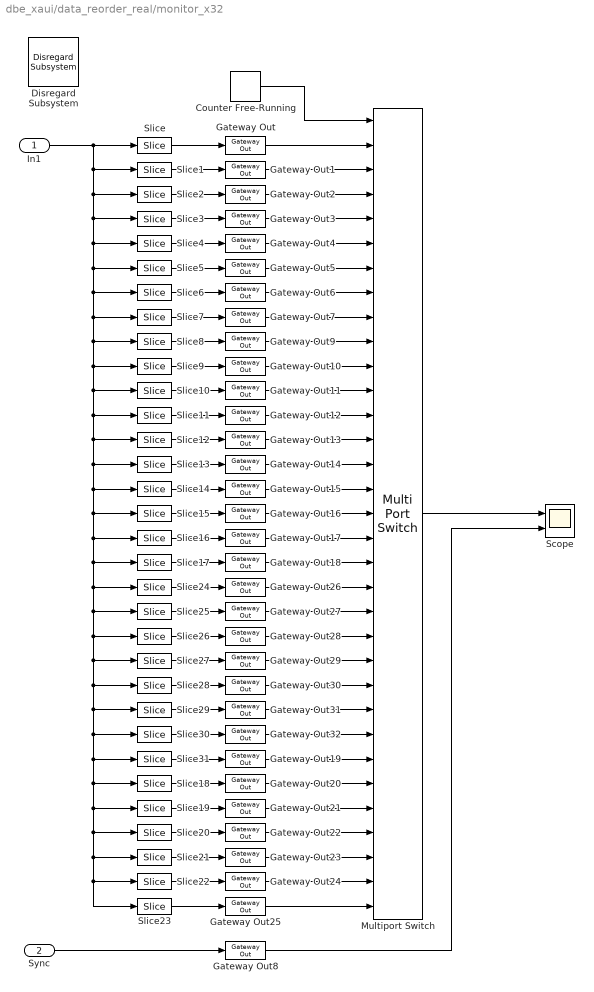
== system dbe_xaui/data_reorder_real/monitor_x32 ==
  In1 : Inport  OutDataType=sfix(16)  OutScaling=2^0
  Sync : Inport  Port=2  OutDataType=sfix(16)  OutScaling=2^0
  Counter Free-Running : simulink/Sources/Counter Free-Running  SystemSampleTime=-1  NumBits=5  tsamp=1/32
  Disregard Subsystem : xbsIndex_r4/Disregard Subsystem  Tag=discardX  block_type=disregard
      settings: block_version=11.3
  Gateway Out{,1..32} (33 alike) : xbsIndex_r4/Gateway Out  hdl_port=off  timing_constraint=None  locs_specified=off  LOCs={}  block_type=gatewayout
      settings: infoedit="Gateway out block.  Converts Xilinx fixed point inputs into ouputs of type Simulink integer, double, or fixed point.<br><br>Hardware notes:  In hardware these blocks become top level output ports or are discarded, depending on how they are configured."  xl_area=[0, 0, 0, 0, 0, 0, 0]  block_version=11.3
  Multiport Switch : MultiPortSwitch  Inputs=32  zeroidx=on  SampleTime=1/32
  Scope : Scope  NumInputPorts=2
      settings: YMin=-5~-5  YMax=5~5
  Slice : xbsIndex_r4/Slice  nbits=sample_width  boolean_output=off  mode=Lower Bit Location + Width  bit1=sample_width*0  base1=MSB of Input  bit0=sample_width*7  base0=LSB of Input  dbl_ovrd=off  block_type=slice
      settings: block_version=11.3
  Slice1 : xbsIndex_r4/Slice  nbits=sample_width  boolean_output=off  mode=Lower Bit Location + Width  bit1=-2*sample_width  base1=MSB of Input  bit0=sample_width*6  base0=LSB of Input  dbl_ovrd=off  block_type=slice
      settings: block_version=11.3
  Slice10 : xbsIndex_r4/Slice  nbits=sample_width  boolean_output=off  mode=Lower Bit Location + Width  bit1=sample_width*21  base1=MSB of Input  bit0=sample_width*21  base0=LSB of Input  dbl_ovrd=off  block_type=slice
      settings: block_version=11.3
  Slice11 : xbsIndex_r4/Slice  nbits=sample_width  boolean_output=off  mode=Lower Bit Location + Width  bit1=-22*sample_width  base1=MSB of Input  bit0=sample_width*20  base0=LSB of Input  dbl_ovrd=off  block_type=slice
      settings: block_version=11.3
  Slice12 : xbsIndex_r4/Slice  nbits=sample_width  boolean_output=off  mode=Lower Bit Location + Width  bit1=-24*sample_width  base1=MSB of Input  bit0=sample_width*31  base0=LSB of Input  dbl_ovrd=off  block_type=slice
      settings: block_version=11.3
  Slice13 : xbsIndex_r4/Slice  nbits=sample_width  boolean_output=off  mode=Lower Bit Location + Width  bit1=-26*sample_width  base1=MSB of Input  bit0=sample_width*30  base0=LSB of Input  dbl_ovrd=off  block_type=slice
      settings: block_version=11.3
  Slice14 : xbsIndex_r4/Slice  nbits=sample_width  boolean_output=off  mode=Lower Bit Location + Width  bit1=-28*sample_width  base1=MSB of Input  bit0=sample_width*29  base0=LSB of Input  dbl_ovrd=off  block_type=slice
      settings: block_version=11.3
  Slice15 : xbsIndex_r4/Slice  nbits=sample_width  boolean_output=off  mode=Lower Bit Location + Width  bit1=-30*sample_width  base1=MSB of Input  bit0=sample_width*28  base0=LSB of Input  dbl_ovrd=off  block_type=slice
      settings: block_version=11.3
  Slice16 : xbsIndex_r4/Slice  nbits=sample_width  boolean_output=off  mode=Lower Bit Location + Width  bit1=-1*sample_width  base1=MSB of Input  bit0=sample_width*3  base0=LSB of Input  dbl_ovrd=off  block_type=slice
      settings: block_version=11.3
  Slice17 : xbsIndex_r4/Slice  nbits=sample_width  boolean_output=off  mode=Lower Bit Location + Width  bit1=-3*sample_width  base1=MSB of Input  bit0=sample_width*2  base0=LSB of Input  dbl_ovrd=off  block_type=slice
      settings: block_version=11.3
  Slice18 : xbsIndex_r4/Slice  nbits=sample_width  boolean_output=off  mode=Lower Bit Location + Width  bit1=-21*sample_width  base1=MSB of Input  bit0=sample_width*17  base0=LSB of Input  dbl_ovrd=off  block_type=slice
      settings: block_version=11.3
  Slice19 : xbsIndex_r4/Slice  nbits=sample_width  boolean_output=off  mode=Lower Bit Location + Width  bit1=-23*sample_width  base1=MSB of Input  bit0=sample_width*16  base0=LSB of Input  dbl_ovrd=off  block_type=slice
      settings: block_version=11.3
  Slice2 : xbsIndex_r4/Slice  nbits=sample_width  boolean_output=off  mode=Lower Bit Location + Width  bit1=-4*sample_width  base1=MSB of Input  bit0=sample_width*5  base0=LSB of Input  dbl_ovrd=off  block_type=slice
      settings: block_version=11.3
  Slice20 : xbsIndex_r4/Slice  nbits=sample_width  boolean_output=off  mode=Lower Bit Location + Width  bit1=-25*sample_width  base1=MSB of Input  bit0=sample_width*27  base0=LSB of Input  dbl_ovrd=off  block_type=slice
      settings: block_version=11.3
  Slice21 : xbsIndex_r4/Slice  nbits=sample_width  boolean_output=off  mode=Lower Bit Location + Width  bit1=-27*sample_width  base1=MSB of Input  bit0=sample_width*26  base0=LSB of Input  dbl_ovrd=off  block_type=slice
      settings: block_version=11.3
  Slice22 : xbsIndex_r4/Slice  nbits=sample_width  boolean_output=off  mode=Lower Bit Location + Width  bit1=-29*sample_width  base1=MSB of Input  bit0=sample_width*25  base0=LSB of Input  dbl_ovrd=off  block_type=slice
      settings: block_version=11.3
  Slice23 : xbsIndex_r4/Slice  nbits=sample_width  boolean_output=off  mode=Lower Bit Location + Width  bit1=-31*sample_width  base1=MSB of Input  bit0=sample_width*24  base0=LSB of Input  dbl_ovrd=off  block_type=slice
      settings: block_version=11.3
  Slice24 : xbsIndex_r4/Slice  nbits=sample_width  boolean_output=off  mode=Lower Bit Location + Width  bit1=-5*sample_width  base1=MSB of Input  bit0=sample_width*1  base0=LSB of Input  dbl_ovrd=off  block_type=slice
      settings: block_version=11.3
  Slice25 : xbsIndex_r4/Slice  nbits=sample_width  boolean_output=off  mode=Lower Bit Location + Width  bit1=-7*sample_width  base1=MSB of Input  bit0=sample_width*0  base0=LSB of Input  dbl_ovrd=off  block_type=slice
      settings: block_version=11.3
  Slice26 : xbsIndex_r4/Slice  nbits=sample_width  boolean_output=off  mode=Lower Bit Location + Width  bit1=-9*sample_width  base1=MSB of Input  bit0=sample_width*11  base0=LSB of Input  dbl_ovrd=off  block_type=slice
      settings: block_version=11.3
  Slice27 : xbsIndex_r4/Slice  nbits=sample_width  boolean_output=off  mode=Lower Bit Location + Width  bit1=-11*sample_width  base1=MSB of Input  bit0=sample_width*10  base0=LSB of Input  dbl_ovrd=off  block_type=slice
      settings: block_version=11.3
  Slice28 : xbsIndex_r4/Slice  nbits=sample_width  boolean_output=off  mode=Lower Bit Location + Width  bit1=-13*sample_width  base1=MSB of Input  bit0=sample_width*9  base0=LSB of Input  dbl_ovrd=off  block_type=slice
      settings: block_version=11.3
  Slice29 : xbsIndex_r4/Slice  nbits=sample_width  boolean_output=off  mode=Lower Bit Location + Width  bit1=-15*sample_width  base1=MSB of Input  bit0=sample_width*8  base0=LSB of Input  dbl_ovrd=off  block_type=slice
      settings: block_version=11.3
  Slice3 : xbsIndex_r4/Slice  nbits=sample_width  boolean_output=off  mode=Lower Bit Location + Width  bit1=-6*sample_width  base1=MSB of Input  bit0=sample_width*4  base0=LSB of Input  dbl_ovrd=off  block_type=slice
      settings: block_version=11.3
  Slice30 : xbsIndex_r4/Slice  nbits=sample_width  boolean_output=off  mode=Lower Bit Location + Width  bit1=-17*sample_width  base1=MSB of Input  bit0=sample_width*19  base0=LSB of Input  dbl_ovrd=off  block_type=slice
      settings: block_version=11.3
  Slice31 : xbsIndex_r4/Slice  nbits=sample_width  boolean_output=off  mode=Lower Bit Location + Width  bit1=sample_width*18  base1=MSB of Input  bit0=sample_width*18  base0=LSB of Input  dbl_ovrd=off  block_type=slice
      settings: block_version=11.3
  Slice4 : xbsIndex_r4/Slice  nbits=sample_width  boolean_output=off  mode=Lower Bit Location + Width  bit1=-8*sample_width  base1=MSB of Input  bit0=sample_width*15  base0=LSB of Input  dbl_ovrd=off  block_type=slice
      settings: block_version=11.3
  Slice5 : xbsIndex_r4/Slice  nbits=sample_width  boolean_output=off  mode=Lower Bit Location + Width  bit1=-10*sample_width  base1=MSB of Input  bit0=sample_width*14  base0=LSB of Input  dbl_ovrd=off  block_type=slice
      settings: block_version=11.3
  Slice6 : xbsIndex_r4/Slice  nbits=sample_width  boolean_output=off  mode=Lower Bit Location + Width  bit1=-12*sample_width  base1=MSB of Input  bit0=sample_width*13  base0=LSB of Input  dbl_ovrd=off  block_type=slice
      settings: block_version=11.3
  Slice7 : xbsIndex_r4/Slice  nbits=sample_width  boolean_output=off  mode=Lower Bit Location + Width  bit1=-14*sample_width  base1=MSB of Input  bit0=sample_width*12  base0=LSB of Input  dbl_ovrd=off  block_type=slice
      settings: block_version=11.3
  Slice8 : xbsIndex_r4/Slice  nbits=sample_width  boolean_output=off  mode=Lower Bit Location + Width  bit1=-16*sample_width  base1=MSB of Input  bit0=sample_width*23  base0=LSB of Input  dbl_ovrd=off  block_type=slice
      settings: block_version=11.3
  Slice9 : xbsIndex_r4/Slice  nbits=sample_width  boolean_output=off  mode=Lower Bit Location + Width  bit1=-18*sample_width  base1=MSB of Input  bit0=sample_width*22  base0=LSB of Input  dbl_ovrd=off  block_type=slice
      settings: block_version=11.3
  wires:
  Gateway Out25/1 -> Multiport Switch/33
  Gateway Out24/1 -> Multiport Switch/32
  Gateway Out23/1 -> Multiport Switch/31
  Gateway Out22/1 -> Multiport Switch/30
  Gateway Out21/1 -> Multiport Switch/29
  Gateway Out20/1 -> Multiport Switch/28
  Gateway Out19/1 -> Multiport Switch/27
  Gateway Out32/1 -> Multiport Switch/26
  Gateway Out31/1 -> Multiport Switch/25
  Gateway Out30/1 -> Multiport Switch/24
  Gateway Out29/1 -> Multiport Switch/23
  Gateway Out28/1 -> Multiport Switch/22
  Gateway Out27/1 -> Multiport Switch/21
  Gateway Out26/1 -> Multiport Switch/20
  Gateway Out18/1 -> Multiport Switch/19
  Gateway Out17/1 -> Multiport Switch/18
  Gateway Out16/1 -> Multiport Switch/17
  Gateway Out15/1 -> Multiport Switch/16
  Gateway Out14/1 -> Multiport Switch/15
  Gateway Out13/1 -> Multiport Switch/14
  Gateway Out12/1 -> Multiport Switch/13
  Gateway Out11/1 -> Multiport Switch/12
  Gateway Out10/1 -> Multiport Switch/11
  Gateway Out9/1 -> Multiport Switch/10
  Gateway Out7/1 -> Multiport Switch/9
  Gateway Out6/1 -> Multiport Switch/8
  Gateway Out5/1 -> Multiport Switch/7
  Gateway Out4/1 -> Multiport Switch/6
  Gateway Out3/1 -> Multiport Switch/5
  Gateway Out2/1 -> Multiport Switch/4
  Gateway Out1/1 -> Multiport Switch/3
  Gateway Out/1 -> Multiport Switch/2
  Slice30/1 -> Gateway Out32/1
  Slice31/1 -> Gateway Out19/1
  Slice18/1 -> Gateway Out20/1
  Slice19/1 -> Gateway Out21/1
  Slice20/1 -> Gateway Out22/1
  Slice21/1 -> Gateway Out23/1
  Slice22/1 -> Gateway Out24/1
  Slice23/1 -> Gateway Out25/1
  Slice16/1 -> Gateway Out17/1
  Slice17/1 -> Gateway Out18/1
  Slice24/1 -> Gateway Out26/1
  Slice25/1 -> Gateway Out27/1
  Slice26/1 -> Gateway Out28/1
  Slice27/1 -> Gateway Out29/1
  Slice28/1 -> Gateway Out30/1
  Slice29/1 -> Gateway Out31/1
  Slice8/1 -> Gateway Out9/1
  Slice9/1 -> Gateway Out10/1
  Slice10/1 -> Gateway Out11/1
  Slice11/1 -> Gateway Out12/1
  Slice12/1 -> Gateway Out13/1
  Slice13/1 -> Gateway Out14/1
  Slice14/1 -> Gateway Out15/1
  Slice15/1 -> Gateway Out16/1
  In1/1 -> Slice/1, Slice1/1, Slice2/1, Slice3/1, Slice4/1, Slice5/1, Slice8/1, Slice9/1, Slice10/1, Slice11/1, Slice12/1, Slice13/1, Slice28/1, Slice29/1, Slice23/1, Slice22/1, Slice21/1, Slice20/1, Slice19/1, Slice18/1, Slice31/1, Slice30/1, Slice27/1, Slice26/1, Slice25/1, Slice24/1, Slice17/1, Slice16/1, Slice15/1, Slice14/1, Slice7/1, Slice6/1
  Slice/1 -> Gateway Out/1
  Slice1/1 -> Gateway Out1/1
  Slice2/1 -> Gateway Out2/1
  Slice3/1 -> Gateway Out3/1
  Slice4/1 -> Gateway Out4/1
  Slice5/1 -> Gateway Out5/1
  Slice6/1 -> Gateway Out6/1
  Slice7/1 -> Gateway Out7/1
  Counter Free-Running/1 -> Multiport Switch/1
  Multiport Switch/1 -> Scope/1
  Sync/1 -> Gateway Out8/1
  Gateway Out8/1 -> Scope/2

== system dbe_xaui/data_reorder_real/monitor_x8 ==
  In1 : Inport  OutDataType=sfix(16)  OutScaling=2^0
  Sync : Inport  Port=2  OutDataType=sfix(16)  OutScaling=2^0
  Counter Free-Running : simulink/Sources/Counter Free-Running  SystemSampleTime=-1  NumBits=3  tsamp=1/8
  Disregard Subsystem : xbsIndex_r4/Disregard Subsystem  Tag=discardX  block_type=disregard
      settings: block_version=11.3
  Gateway Out{,1..8} (9 alike) : xbsIndex_r4/Gateway Out  hdl_port=off  timing_constraint=None  locs_specified=off  LOCs={}  block_type=gatewayout
      settings: infoedit="Gateway out block.  Converts Xilinx fixed point inputs into ouputs of type Simulink integer, double, or fixed point.<br><br>Hardware notes:  In hardware these blocks become top level output ports or are discarded, depending on how they are configured."  xl_area=[0, 0, 0, 0, 0, 0, 0]  block_version=11.3
  Multiport Switch : MultiPortSwitch  Inputs=8  zeroidx=on  SampleTime=1/8
  Scope : Scope  NumInputPorts=2
      settings: YMin=-5~-5  YMax=5~5
  Slice : xbsIndex_r4/Slice  nbits=sample_width  boolean_output=off  mode=Upper Bit Location + Width  bit1=0  base1=MSB of Input  bit0=0  base0=LSB of Input  dbl_ovrd=off  block_type=slice
      settings: block_version=11.3
  Slice1 : xbsIndex_r4/Slice  nbits=sample_width  boolean_output=off  mode=Upper Bit Location + Width  bit1=-1*sample_width  base1=MSB of Input  bit0=0  base0=LSB of Input  dbl_ovrd=off  block_type=slice
      settings: block_version=11.3
  Slice2 : xbsIndex_r4/Slice  nbits=sample_width  boolean_output=off  mode=Upper Bit Location + Width  bit1=-2*sample_width  base1=MSB of Input  bit0=0  base0=LSB of Input  dbl_ovrd=off  block_type=slice
      settings: block_version=11.3
  Slice3 : xbsIndex_r4/Slice  nbits=sample_width  boolean_output=off  mode=Upper Bit Location + Width  bit1=-3*sample_width  base1=MSB of Input  bit0=0  base0=LSB of Input  dbl_ovrd=off  block_type=slice
      settings: block_version=11.3
  Slice4 : xbsIndex_r4/Slice  nbits=sample_width  boolean_output=off  mode=Upper Bit Location + Width  bit1=-4*sample_width  base1=MSB of Input  bit0=0  base0=LSB of Input  dbl_ovrd=off  block_type=slice
      settings: block_version=11.3
  Slice5 : xbsIndex_r4/Slice  nbits=sample_width  boolean_output=off  mode=Upper Bit Location + Width  bit1=-5*sample_width  base1=MSB of Input  bit0=0  base0=LSB of Input  dbl_ovrd=off  block_type=slice
      settings: block_version=11.3
  Slice6 : xbsIndex_r4/Slice  nbits=sample_width  boolean_output=off  mode=Upper Bit Location + Width  bit1=-6*sample_width  base1=MSB of Input  bit0=0  base0=LSB of Input  dbl_ovrd=off  block_type=slice
      settings: block_version=11.3
  Slice7 : xbsIndex_r4/Slice  nbits=sample_width  boolean_output=off  mode=Upper Bit Location + Width  bit1=-7*sample_width  base1=MSB of Input  bit0=0  base0=LSB of Input  dbl_ovrd=off  block_type=slice
      settings: block_version=11.3
  wires:
  Gateway Out8/1 -> Scope/2
  Sync/1 -> Gateway Out8/1
  Multiport Switch/1 -> Scope/1
  Gateway Out7/1 -> Multiport Switch/9
  Gateway Out6/1 -> Multiport Switch/8
  Gateway Out5/1 -> Multiport Switch/7
  Gateway Out4/1 -> Multiport Switch/6
  Gateway Out3/1 -> Multiport Switch/5
  Gateway Out2/1 -> Multiport Switch/4
  Gateway Out1/1 -> Multiport Switch/3
  Gateway Out/1 -> Multiport Switch/2
  Counter Free-Running/1 -> Multiport Switch/1
  Slice7/1 -> Gateway Out7/1
  Slice6/1 -> Gateway Out6/1
  Slice5/1 -> Gateway Out5/1
  Slice4/1 -> Gateway Out4/1
  Slice3/1 -> Gateway Out3/1
  Slice2/1 -> Gateway Out2/1
  Slice1/1 -> Gateway Out1/1
  Slice/1 -> Gateway Out/1
  In1/1 -> Slice7/1, Slice6/1, Slice5/1, Slice4/1, Slice3/1, Slice2/1, Slice1/1, Slice/1
